simulink model "wlan_phy_rx_pmd"  (Simulink 7.8)
settings: Solver=VariableStepDiscrete  SolverMode=SingleTasking  StartTime=0.0  StopTime=rx_sim.sim_time  RelTol=1e-3
PostLoadFcn:
  | wlan_phy_rx_init
InitFcn:
  | wlan_phy_rx_init
StartFcn:
  | wlan_phy_rx_init
values from neighbouring files (symbols this model uses):
  barker_seq20 = 0.95 * circshift(barker_seq20, [0 4]) ./ max(abs(barker_seq20))    [wlan_phy_rx_init.m]
  bit_scrambler_lfsr_bytes = sum(bit_scrambler_lfsr_bytes .* repmat(2.^[0:7], 127, 1),2)    [wlan_phy_rx_init.m]
  longCorr_coef_i = [3*real(longCorr_coef)]    [wlan_phy_rx_init.m]
  longCorr_coef_q = [3*imag(longCorr_coef)]    [wlan_phy_rx_init.m]
  MAX_CP_LEN = 32    [wlan_phy_rx_init.m]
  MAX_NCBPS = NCBPS_11n_64QAM    (= 312)    [wlan_phy_rx_init.m]
  MAX_NUM_BYTES = 2^16-1    (= 65535)    [wlan_phy_rx_init.m]
  MAX_NUM_SC = 64    [wlan_phy_rx_init.m]
  MAX_NUM_SYMS = 600    [wlan_phy_rx_init.m]
  NDBPS_11a_BPSK12 = 24    [wlan_phy_rx_init.m]
  preFFT_sampBuff_numSamps = 4*MAX_NUM_SC    (= 256)    [wlan_phy_rx_init.m]
  rx_sim = struct()    [wlan_phy_rx_init.m]
  scr_ind_rev = zeros(1,128)    [wlan_phy_rx_init.m]
undefined symbols (not defined in the file or in its folder): gcbh, mcs_rom_11ag, mcs_rom_11n, wlan_rx_deinterleave_rom_gen, xlBlockLoadCallback, xlGetNormalizedPeriod, xlipgetpartsetting
libraries: xbsIndex_r4 x4943, xbsEDKLib_r4 x1
(part 12 of 28)

== system wlan_phy_rx_pmd/FCS & Pkt Buf/Pkt Buf Interface/BRAM IF 64b ==
  note: Word Addr
      | (1K words max)
  "byte " : Inport
  byte_valid : Inport  Port=2
  byte_ind : Inport  Port=3
  Pkt Buf Sel : Inport  Port=4
  9LSB + 3 : xbsIndex_r4/Slice  nbits=9  boolean_output=off  mode=Lower Bit Location + Width  bit1=2  base1=MSB of Input  bit0=3  base0=LSB of Input  dbl_ovrd=off  block_type=slice
      settings: block_version=8.2.02
  AddSub1 : xbsIndex_r4/AddSub  mode=Addition  use_carryin=off  use_carryout=off  en=off  latency=0  precision=Full  arith_type=Unsigned  n_bits=15  bin_pt=0  quantization=Truncate  overflow=Wrap  dbl_ovrd=off  use_behavioral_HDL=off  hw_selection=Fabric  pipelined=off  block_type=addsub
  AddSub2 : xbsIndex_r4/AddSub  mode=Addition  use_carryin=off  use_carryout=off  en=off  latency=0  precision=Full  arith_type=Unsigned  n_bits=15  bin_pt=0  quantization=Truncate  overflow=Wrap  dbl_ovrd=off  use_behavioral_HDL=off  hw_selection=Fabric  pipelined=off  block_type=addsub
  BRAM I/O : Subsystem
  BRAM WEn Decoding : Subsystem
  Concat : xbsIndex_r4/Concat  num_inputs=4  dbl_ovrd=off  block_type=concat
      settings: block_version=8.2.02
  Concat1 : xbsIndex_r4/Concat  num_inputs=4  dbl_ovrd=off  block_type=concat
      settings: block_version=8.2.02
  Concat{2..4,6..7} (5 alike) : xbsIndex_r4/Concat  num_inputs=2  dbl_ovrd=off  block_type=concat
      settings: block_version=8.2.02
  Concat5 : xbsIndex_r4/Concat  num_inputs=4  dbl_ovrd=off  block_type=concat
      settings: block_version=8.2.02
  Constant1 : xbsIndex_r4/Constant  const=0  gui_display_data_type=Fixed-point  arith_type=Unsigned  n_bits=2  bin_pt=0  preci_type=Single  exp_width=8  frac_width=24  explicit_period=off  period=1  equ=P=C  opselect=C  inp2=PCIN>>17  opr=+  inp1=P  carry=CIN  dbl_ovrd=off  block_type=constant
  Constant2 : xbsIndex_r4/Constant  const=0  gui_display_data_type=Fixed-point  arith_type=Unsigned  n_bits=4  bin_pt=0  preci_type=Single  exp_width=8  frac_width=24  explicit_period=off  period=1  equ=P=C  opselect=C  inp2=PCIN>>17  opr=+  inp1=P  carry=CIN  dbl_ovrd=off  block_type=constant
  Constant3 : xbsIndex_r4/Constant  const=0  gui_display_data_type=Fixed-point  arith_type=Unsigned  n_bits=16  bin_pt=0  preci_type=Single  exp_width=8  frac_width=24  explicit_period=on  period=1  equ=P=C  opselect=C  inp2=PCIN>>17  opr=+  inp1=P  carry=CIN  dbl_ovrd=off  block_type=constant
      settings: block_version=8.2.02
  Constant4 : xbsIndex_r4/Constant  const=0  gui_display_data_type=Fixed-point  arith_type=Unsigned  n_bits=3  bin_pt=0  preci_type=Single  exp_width=8  frac_width=24  explicit_period=off  period=1  equ=P=C  opselect=C  inp2=PCIN>>17  opr=+  inp1=P  carry=CIN  dbl_ovrd=off  block_type=constant
  Constant5 : xbsIndex_r4/Constant  const=0  gui_display_data_type=Fixed-point  arith_type=Unsigned  n_bits=3  bin_pt=0  preci_type=Single  exp_width=8  frac_width=24  explicit_period=on  period=1  equ=P=C  opselect=C  inp2=PCIN>>17  opr=+  inp1=P  carry=CIN  dbl_ovrd=off  block_type=constant
      settings: block_version=8.2.02
  Constant7 : xbsIndex_r4/Constant  const=0  gui_display_data_type=Fixed-point  arith_type=Unsigned  n_bits=3  bin_pt=0  preci_type=Single  exp_width=8  frac_width=24  explicit_period=off  period=1  equ=P=C  opselect=C  inp2=PCIN>>17  opr=+  inp1=P  carry=CIN  dbl_ovrd=off  block_type=constant
  Convert : xbsIndex_r4/Convert  gui_display_data_type=Fixed-point  arith_type=Unsigned  n_bits=8  bin_pt=0  float_type=Single  exp_bits=8  fraction_bits=24  quantization=Truncate  overflow=Wrap  en=off  latency=0  dbl_ovrd=off  pipeline=off  block_type=convert
  Display : Display
  Gateway Out{1..12} (12 alike) : xbsIndex_r4/Gateway Out  inherit_from_input=off  hdl_port=off  timing_constraint=None  locs_specified=off  LOCs={}  UseAsDAC=off  DACChannel='1'  block_type=gatewayout
  LSB : xbsIndex_r4/Slice  nbits=1  boolean_output=off  mode=Lower Bit Location + Width  bit1=0  base1=MSB of Input  bit0=0  base0=LSB of Input  dbl_ovrd=off  block_type=slice
      settings: block_version=8.2.02
  Logical : xbsIndex_r4/Logical  logical_function=AND  inputs=2  en=off  latency=0  precision=Full  arith_type=Unsigned  n_bits=16  bin_pt=0  align_bp=on  dbl_ovrd=off  block_type=logical
      settings: block_version=8.2.02
  Logical4 : xbsIndex_r4/Logical  logical_function=XOR  inputs=2  en=off  latency=0  precision=Full  arith_type=Unsigned  n_bits=16  bin_pt=0  align_bp=on  dbl_ovrd=off  block_type=logical
  Mux{,1..3} (4 alike) : xbsIndex_r4/Mux  inputs=2  en=off  latency=0  precision=Full  arith_type=Unsigned  n_bits=16  bin_pt=14  quantization=Truncate  overflow=Wrap  dbl_ovrd=off  block_type=mux
  Pkt Buf Addr : Scope  NumInputPorts=8
      settings: TimeRange=10000  YMin=0~0~0~0~0~-1~-1~-1  YMax=17.5~150~17.5~1~4.5e+009~1~1~1  SaveName=ScopeData27
  To Workspace : ToWorkspace  VariableName=pkt_buf_bram_data  SampleTime=-1  SaveFormat=Array
  To Workspace1 : ToWorkspace  VariableName=pkt_buf_bram_addr  SampleTime=-1  SaveFormat=Array
  To Workspace2 : ToWorkspace  VariableName=pkt_buf_bram_wen  SampleTime=-1  SaveFormat=Array
  wires:
  Gateway Out4/1 -> Pkt Buf Addr/4  "Rx Byte Valid"
  9LSB + 3/1 -> Concat1/3
  byte_valid/1 -> BRAM WEn Decoding/2(byte_valid), Gateway Out4/1
  AddSub2/1 -> Mux1/2, BRAM WEn Decoding/1(byte_addr), Gateway Out2/1
  Pkt Buf Sel/1 -> Concat1/2
  "byte "/1 -> Concat/1, Concat/2, Concat/3, Concat/4
  Gateway Out2/1 -> Pkt Buf Addr/3  "Rx Byte Index"
  Gateway Out1/1 -> Pkt Buf Addr/2  "BRAM WEN"
  Gateway Out10/1 -> Pkt Buf Addr/1  "BRAM Addr"
  Constant3/1 -> Concat1/1
  Constant5/1 -> Concat1/4
  Concat1/1 -> BRAM I/O/2(Addr[31:0]), Gateway Out10/1, Gateway Out11/1
  BRAM WEn Decoding/1(WrEn[7:0]) -> Mux/2
  byte_ind/1 -> AddSub2/1
  Concat2/1 -> AddSub2/2
  [regRx_PktBuf_Rx_Addr_Offset] -> Concat2/1
  Constant7/1 -> Concat2/2
  Gateway Out3/1 -> Pkt Buf Addr/8  "H Est Valid"
  Mux2/1 -> Concat3/1, Concat3/2, Gateway Out7/1, Gateway Out9/1
  Concat/1 -> Mux2/2
  [CHAN_EST_IND] -> Concat4/1, LSB/1, Gateway Out5/1
  Constant1/1 -> Concat4/2
  Mux1/1 -> 9LSB + 3/1
  Logical/1 -> Mux2/1, Mux1/1, Mux/1
  Mux3/1 -> Mux/3, Gateway Out6/1
  [CHAN_EST_VALID] -> Concat5/1, Concat5/2, Concat5/3, Concat5/4, Gateway Out3/1
  Concat5/1 -> Concat6/1, Convert/1
  Constant2/1 -> Concat6/2
  [regRx_Record_Chan_Est_Pkt_Buf] -> Logical/1
  [CHAN_EST_VALID] -> Logical/2
  Concat4/1 -> AddSub1/2
  Concat7/1 -> AddSub1/1
  [regRx_PktBuf_Rx_H_Est_Offset] -> Concat7/1, Gateway Out8/1
  Constant4/1 -> Concat7/2
  AddSub1/1 -> Mux1/3
  Concat3/1 -> BRAM I/O/1(Dout[63:0])
  Mux/1 -> BRAM I/O/3(WEn[7:0]), Gateway Out1/1, Gateway Out12/1
  Concat6/1 -> Mux3/3
  Convert/1 -> Mux3/2
  [regRx_PKT_BUF_H_EST_ORDER_SWAP] -> Logical4/1
  LSB/1 -> Logical4/2
  Logical4/1 -> Mux3/1
  Gateway Out5/1 -> Pkt Buf Addr/7  "H Est Ind"
  Gateway Out6/1 -> Pkt Buf Addr/6  "H Est WrEn"
  [CHAN_EST_IQ_32b] -> Mux2/3
  Gateway Out7/1 -> Pkt Buf Addr/5  "Dout 32"
  Gateway Out8/1 -> Display/1
  Gateway Out11/1 -> To Workspace1/1
  Gateway Out9/1 -> To Workspace/1
  Gateway Out12/1 -> To Workspace2/1

== system wlan_phy_rx_pmd/FCS & Pkt Buf/Pkt Buf Interface/DSSS Addr Calc ==
  note: DSSS Byte Index Rx -> Memory mapping
      |
      | R=Rx byte index
      | M=Memory byte index
      |
      | R  M
      | 0  0   SIGNAL[0]
      | 1  1   SERVICE[0]
      | 2  2   LENGTH[0]
      | 3  3   LENGTH[1]
      | 4  4   CRC16[0]
      | 5  5   CRC16[1]
      |    6   no write
      |   ...
      |    15  no write
      | 6  16  MPDU[0]
      | 7  17  MPDU[1]
      | 8  18  MPDU[2]
      | ...
  R : Inport
  AddSub1 : xbsIndex_r4/AddSub  mode=Addition  use_carryin=off  use_carryout=off  en=off  latency=0  precision=Full  arith_type=Unsigned  n_bits=3  bin_pt=0  quantization=Truncate  overflow=Wrap  dbl_ovrd=off  use_behavioral_HDL=off  hw_selection=Fabric  pipelined=off  block_type=addsub
  Constant1 : xbsIndex_r4/Constant  const=5  gui_display_data_type=Fixed-point  arith_type=Unsigned  n_bits=3  bin_pt=0  preci_type=Single  exp_width=8  frac_width=24  explicit_period=off  period=1  equ=P=C  opselect=C  inp2=PCIN>>17  opr=+  inp1=P  carry=CIN  dbl_ovrd=off  block_type=constant
  Constant3 : xbsIndex_r4/Constant  const=10  gui_display_data_type=Fixed-point  arith_type=Unsigned  n_bits=4  bin_pt=0  preci_type=Single  exp_width=8  frac_width=24  explicit_period=off  period=1  equ=P=C  opselect=C  inp2=PCIN>>17  opr=+  inp1=P  carry=CIN  dbl_ovrd=off  block_type=constant
  Mux5 : xbsIndex_r4/Mux  inputs=2  en=off  latency=0  precision=Full  arith_type=Unsigned  n_bits=16  bin_pt=14  quantization=Truncate  overflow=Wrap  dbl_ovrd=off  block_type=mux
  Relational : xbsIndex_r4/Relational  mode=a<=b  en=off  latency=0  dbl_ovrd=off  block_type=relational
  M : Outport
  wires:
  Mux5/1 -> M/1
  Constant1/1 -> Relational/2
  Relational/1 -> Mux5/1
  R/1 -> Relational/1, AddSub1/1, Mux5/3
  Constant3/1 -> AddSub1/2
  AddSub1/1 -> Mux5/2

== system wlan_phy_rx_pmd/FCS & Pkt Buf/Pkt Buf Interface/OFDM Addr Calc ==
  note: OFDM Byte Index Rx -> Memory mapping
      | 11a/g Rx
      |
      | R=Rx byte index
      | M=Memory byte index
      |
      | R  M
      | 0  0   SIGNAL[0]
      | 1  1   SIGNAL[1]
      | 2  2   SIGNAL[2]
      | 3  3   SERVICE[0]
      | 4  4   SERVICE[1]
      |    5   no write
      |    ...
      |    15   no write
      | 5  16  MPDU[0]
      | 6  17  MPDU[1]
      | ...
  note: OFDM Byte Index Rx -> Memory mapping
      | 11n/ac Rx
      |
      | R=Rx byte index
      | M=Memory byte index
      |
      | R  M
      | 0  0   SIGNAL[0]
      | 1  1   SIGNAL[1]
      | 2  2   SIGNAL[2]
      | 3  3   HT-SIG[0]
      | 4  4   HT-SIG[1]
      | 5  5   HT-SIG[2]
      | 6  6   HT-SIG[3]
      | 7  7   HT-SIG[4]
      | 8  8   HT-SIG[5]
      | 9  9   SERVICE[0]
      | 10 10  SERVICE[1]
      |    11  no write
      | ...
      |    15  no write
      | 11 16  MAC Payload[0]
      | 12 17  MAC Payload[1]
      | ...
  R : Inport
  AddSub1 : xbsIndex_r4/AddSub  mode=Addition  use_carryin=off  use_carryout=off  en=off  latency=0  precision=Full  arith_type=Unsigned  n_bits=3  bin_pt=0  quantization=Truncate  overflow=Wrap  dbl_ovrd=off  use_behavioral_HDL=off  hw_selection=Fabric  pipelined=off  block_type=addsub
  Constant1 : xbsIndex_r4/Constant  const=4  gui_display_data_type=Fixed-point  arith_type=Unsigned  n_bits=3  bin_pt=0  preci_type=Single  exp_width=8  frac_width=24  explicit_period=off  period=1  equ=P=C  opselect=C  inp2=PCIN>>17  opr=+  inp1=P  carry=CIN  dbl_ovrd=off  block_type=constant
  Constant2 : xbsIndex_r4/Constant  const=11  gui_display_data_type=Fixed-point  arith_type=Unsigned  n_bits=4  bin_pt=0  preci_type=Single  exp_width=8  frac_width=24  explicit_period=off  period=1  equ=P=C  opselect=C  inp2=PCIN>>17  opr=+  inp1=P  carry=CIN  dbl_ovrd=off  block_type=constant
  Constant3 : xbsIndex_r4/Constant  const=10  gui_display_data_type=Fixed-point  arith_type=Unsigned  n_bits=4  bin_pt=0  preci_type=Single  exp_width=8  frac_width=24  explicit_period=off  period=1  equ=P=C  opselect=C  inp2=PCIN>>17  opr=+  inp1=P  carry=CIN  dbl_ovrd=off  block_type=constant
  Constant4 : xbsIndex_r4/Constant  const=5  gui_display_data_type=Fixed-point  arith_type=Unsigned  n_bits=3  bin_pt=0  preci_type=Single  exp_width=8  frac_width=24  explicit_period=off  period=1  equ=P=C  opselect=C  inp2=PCIN>>17  opr=+  inp1=P  carry=CIN  dbl_ovrd=off  block_type=constant
  Mux1 : xbsIndex_r4/Mux  inputs=2  en=off  latency=0  precision=Full  arith_type=Unsigned  n_bits=16  bin_pt=14  quantization=Truncate  overflow=Wrap  dbl_ovrd=off  block_type=mux
  Mux2 : xbsIndex_r4/Mux  inputs=2  en=off  latency=0  precision=Full  arith_type=Unsigned  n_bits=16  bin_pt=14  quantization=Truncate  overflow=Wrap  dbl_ovrd=off  block_type=mux
  Mux5 : xbsIndex_r4/Mux  inputs=2  en=off  latency=0  precision=Full  arith_type=Unsigned  n_bits=16  bin_pt=14  quantization=Truncate  overflow=Wrap  dbl_ovrd=off  block_type=mux
  Relational : xbsIndex_r4/Relational  mode=a<=b  en=off  latency=0  dbl_ovrd=off  block_type=relational
  M : Outport
  wires:
  Mux2/1 -> AddSub1/2
  R/1 -> Relational/1, AddSub1/1, Mux5/3
  Relational/1 -> Mux5/1
  Mux5/1 -> M/1
  AddSub1/1 -> Mux5/2
  [RX_PHY_MODE_11N_AC] -> Mux1/1, Mux2/1
  Constant1/1 -> Mux1/2
  Mux1/1 -> Relational/2
  Constant2/1 -> Mux2/2
  Constant3/1 -> Mux1/3
  Constant4/1 -> Mux2/3

== system wlan_phy_rx_pmd/FCS & Pkt Buf/Pkt Buf Interface/Rx Data MAC Outputs ==
  note: Byte index output to MAC is
      | indexed so 0 = MPDU start
      | (0x10 into pkt buffer)
  note: Outputs to MAC hw
  "Byte " : Inport
  Byte Valid : Inport  Port=2
  Byte Ind : Inport  Port=3
  AddSub1 : xbsIndex_r4/AddSub  mode=Subtraction  use_carryin=off  use_carryout=off  en=off  latency=0  precision=User Defined  arith_type=Unsigned  n_bits=ceil(log2(MAX_NUM_BYTES))  bin_pt=0  quantization=Truncate  overflow=Saturate  dbl_ovrd=off  use_behavioral_HDL=off  hw_selection=Fabric  pipelined=off  block_type=addsub
  Constant4 : xbsIndex_r4/Constant  const=16  gui_display_data_type=Fixed-point  arith_type=Unsigned  n_bits=5  bin_pt=0  preci_type=Single  exp_width=8  frac_width=24  explicit_period=off  period=1  equ=P=C  opselect=C  inp2=PCIN>>17  opr=+  inp1=P  carry=CIN  dbl_ovrd=off  block_type=constant
  Logical4 : xbsIndex_r4/Logical  logical_function=AND  inputs=2  en=off  latency=0  precision=Full  arith_type=Unsigned  n_bits=16  bin_pt=0  align_bp=on  dbl_ovrd=off  block_type=logical
  Relational2 : xbsIndex_r4/Relational  mode=a>=b  en=off  latency=0  dbl_ovrd=off  block_type=relational
  tag scope: CUR_MPDU_BYTE=global, CUR_MPDU_BYTE_INDEX=global, CUR_MPDU_BYTE_VALID=global
  wires:
  Logical4/1 -> [CUR_MPDU_BYTE_VALID]
  Relational2/1 -> Logical4/1
  AddSub1/1 -> [CUR_MPDU_BYTE_INDEX]
  Constant4/1 -> AddSub1/2, Relational2/2
  Byte Ind/1 -> AddSub1/1, Relational2/1
  Byte Valid/1 -> Logical4/2
  "Byte "/1 -> [CUR_MPDU_BYTE]

== system wlan_phy_rx_pmd/FFT/Reset Gen/S-R Latch1 ==
  S : Inport
  R : Inport  Port=2
  Constant1 : xbsIndex_r4/Constant  const=1  gui_display_data_type=Fixed-point  arith_type=Boolean  n_bits=1  bin_pt=0  preci_type=Single  exp_width=8  frac_width=24  explicit_period=off  period=1  equ=P=C  opselect=C  inp2=PCIN>>17  opr=+  inp1=P  carry=CIN  dbl_ovrd=off  block_type=constant
      settings: block_version=VER_STRING_GOES_HERE
  Convert1 : xbsIndex_r4/Convert  gui_display_data_type=Boolean  arith_type=Boolean  n_bits=1  bin_pt=0  float_type=Single  exp_bits=8  fraction_bits=24  quantization=Truncate  overflow=Wrap  en=off  latency=0  dbl_ovrd=off  pipeline=off  block_type=convert
  Convert2 : xbsIndex_r4/Convert  gui_display_data_type=Boolean  arith_type=Boolean  n_bits=1  bin_pt=0  float_type=Single  exp_bits=8  fraction_bits=24  quantization=Truncate  overflow=Wrap  en=off  latency=0  dbl_ovrd=off  pipeline=off  block_type=convert
  Register2 : xbsIndex_r4/Register  init=0  rst=on  en=on  dbl_ovrd=off  block_type=register
      settings: xl_area=[1 1 0 0 0 0 0]  block_version=VER_STRING_GOES_HERE
  Q : Outport
  wires:
  S/1 -> Convert2/1
  Register2/1 -> Q/1
  R/1 -> Convert1/1
  Constant1/1 -> Register2/1
  Convert1/1 -> Register2/2
  Convert2/1 -> Register2/3

== system wlan_phy_rx_pmd/FFT/Sym Count/S-R Latch1 ==
  S : Inport
  R : Inport  Port=2
  Constant1 : xbsIndex_r4/Constant  const=1  gui_display_data_type=Fixed-point  arith_type=Boolean  n_bits=1  bin_pt=0  preci_type=Single  exp_width=8  frac_width=24  explicit_period=off  period=1  equ=P=C  opselect=C  inp2=PCIN>>17  opr=+  inp1=P  carry=CIN  dbl_ovrd=off  block_type=constant
      settings: block_version=VER_STRING_GOES_HERE
  Convert1 : xbsIndex_r4/Convert  gui_display_data_type=Boolean  arith_type=Boolean  n_bits=1  bin_pt=0  float_type=Single  exp_bits=8  fraction_bits=24  quantization=Truncate  overflow=Wrap  en=off  latency=0  dbl_ovrd=off  pipeline=off  block_type=convert
  Convert2 : xbsIndex_r4/Convert  gui_display_data_type=Boolean  arith_type=Boolean  n_bits=1  bin_pt=0  float_type=Single  exp_bits=8  fraction_bits=24  quantization=Truncate  overflow=Wrap  en=off  latency=0  dbl_ovrd=off  pipeline=off  block_type=convert
  Register2 : xbsIndex_r4/Register  init=0  rst=on  en=on  dbl_ovrd=off  block_type=register
      settings: xl_area=[1 1 0 0 0 0 0]  block_version=VER_STRING_GOES_HERE
  Q : Outport
  wires:
  S/1 -> Convert2/1
  Register2/1 -> Q/1
  R/1 -> Convert1/1
  Constant1/1 -> Register2/1
  Convert1/1 -> Register2/2
  Convert2/1 -> Register2/3

== system wlan_phy_rx_pmd/Inputs/RF A Inputs/RF A RSSI Sum ==
  RSSI : Inport
  Down Sample6 : xbsIndex_r4/Down Sample  sample_ratio=16  sample_phase="Last Value of Frame  (most efficient)"  en=off  latency=1  dbl_ovrd=off  block_type=dsamp
      settings: block_version=VER_STRING_GOES_HERE
  RSSI DET Debug Out : Subsystem
  Running Sum : Subsystem
  Sum : Outport
  wires:
  [RX_GLOBAL_RESET] -> Down Sample6/1
  RSSI/1 -> Running Sum/1(RSSI), RSSI DET Debug Out/2(Raw RSSI)
  Down Sample6/1 -> Running Sum/2(reset)
  Running Sum/1(avg) -> Sum/1, RSSI DET Debug Out/1(Avg RSSI)

== system wlan_phy_rx_pmd/Inputs/RF B Inputs/RF B RSSI Sum ==
  RSSI : Inport
  Down Sample6 : xbsIndex_r4/Down Sample  sample_ratio=16  sample_phase="Last Value of Frame  (most efficient)"  en=off  latency=1  dbl_ovrd=off  block_type=dsamp
      settings: block_version=VER_STRING_GOES_HERE
  Running Sum : Subsystem
  Sum : Outport
  wires:
  Running Sum/1(avg) -> Sum/1
  Down Sample6/1 -> Running Sum/2(reset)
  RSSI/1 -> Running Sum/1(RSSI)
  [RX_GLOBAL_RESET] -> Down Sample6/1

== system wlan_phy_rx_pmd/Inputs/RF C Inputs/RF C RSSI Sum ==
  RSSI : Inport
  Down Sample6 : xbsIndex_r4/Down Sample  sample_ratio=16  sample_phase="Last Value of Frame  (most efficient)"  en=off  latency=1  dbl_ovrd=off  block_type=dsamp
      settings: block_version=VER_STRING_GOES_HERE
  Running Sum : Subsystem
  Sum : Outport
  wires:
  Running Sum/1(avg) -> Sum/1
  Down Sample6/1 -> Running Sum/2(reset)
  RSSI/1 -> Running Sum/1(RSSI)
  [RX_GLOBAL_RESET] -> Down Sample6/1

== system wlan_phy_rx_pmd/Inputs/RF D Inputs/RF D RSSI Sum ==
  RSSI : Inport
  Down Sample6 : xbsIndex_r4/Down Sample  sample_ratio=16  sample_phase="Last Value of Frame  (most efficient)"  en=off  latency=1  dbl_ovrd=off  block_type=dsamp
      settings: block_version=VER_STRING_GOES_HERE
  Running Sum : Subsystem
  Sum : Outport
  wires:
  [RX_GLOBAL_RESET] -> Down Sample6/1
  RSSI/1 -> Running Sum/1(RSSI)
  Down Sample6/1 -> Running Sum/2(reset)
  Running Sum/1(avg) -> Sum/1

== system wlan_phy_rx_pmd/MAC I/O/Pulse Stretch1/S-R Latch ==
  S : Inport
  R : Inport  Port=2
  Constant1 : xbsIndex_r4/Constant  const=1  gui_display_data_type=Boolean  arith_type=Boolean  n_bits=1  bin_pt=0  preci_type=Single  exp_width=8  frac_width=24  explicit_period=off  period=1  equ=P=C  opselect=C  inp2=PCIN>>17  opr=+  inp1=P  carry=CIN  dbl_ovrd=off  block_type=constant
      settings: block_version=VER_STRING_GOES_HERE
  Convert1 : xbsIndex_r4/Convert  gui_display_data_type=Boolean  arith_type=Boolean  n_bits=1  bin_pt=0  float_type=Single  exp_bits=8  fraction_bits=24  quantization=Truncate  overflow=Wrap  en=off  latency=0  dbl_ovrd=off  pipeline=off  block_type=convert
  Convert2 : xbsIndex_r4/Convert  gui_display_data_type=Boolean  arith_type=Boolean  n_bits=1  bin_pt=0  float_type=Single  exp_bits=8  fraction_bits=24  quantization=Truncate  overflow=Wrap  en=off  latency=0  dbl_ovrd=off  pipeline=off  block_type=convert
  Register2 : xbsIndex_r4/Register  init=0  rst=on  en=on  dbl_ovrd=off  block_type=register
      settings: xl_area=[1 1 0 0 0 0 0]  block_version=VER_STRING_GOES_HERE
  Q : Outport
  wires:
  Convert2/1 -> Register2/3
  Convert1/1 -> Register2/2
  Constant1/1 -> Register2/1
  R/1 -> Convert1/1
  Register2/1 -> Q/1
  S/1 -> Convert2/1

== system wlan_phy_rx_pmd/Sync & Antenna Sel/Antenna Selection/Ant Sel Logic ==
  note: [0 0] = RF A
      | [0 1] = RF B
      | [1 0] = RF C
      | [1 1] = RF D
  note: [1 1] = RF A
      | [1 0] = RF B
      | [0 1] = RF C
      | [0 0] = RF D
  G A : Inport
  G B : Inport  Port=2
  G C : Inport  Port=3
  G D : Inport  Port=4
  Concat : xbsIndex_r4/Concat  num_inputs=2  dbl_ovrd=off  block_type=concat
  Inverter2 : xbsIndex_r4/Inverter  en=off  latency=0  dbl_ovrd=off  block_type=inv
  Mux{,1..3} (4 alike) : xbsIndex_r4/Mux  inputs=2  en=off  latency=0  precision=Full  arith_type=Unsigned  n_bits=16  bin_pt=14  quantization=Truncate  overflow=Wrap  dbl_ovrd=off  block_type=mux
  RF A En Mask : Subsystem
  RF B En Mask : Subsystem
  RF C En Mask : Subsystem
  RF D En Mask : Subsystem
  Relational : xbsIndex_r4/Relational  mode=a<=b  en=off  latency=0  dbl_ovrd=off  block_type=relational
  Relational1 : xbsIndex_r4/Relational  mode=a<=b  en=off  latency=0  dbl_ovrd=off  block_type=relational
  Relational2 : xbsIndex_r4/Relational  mode=a<=b  en=off  latency=0  dbl_ovrd=off  block_type=relational
  Ant Sel : Outport
  wires:
  RF D En Mask/1(" GD") -> Relational1/2, Mux1/2
  RF C En Mask/1(" GC") -> Relational1/1, Mux1/3
  RF B En Mask/1(" GB") -> Relational/2, Mux/2
  RF A En Mask/1(" GA") -> Relational/1, Mux/3
  Relational/1 -> Mux/1, Mux2/3
  Mux/1 -> Relational2/1
  Relational1/1 -> Mux1/1, Mux2/2
  Mux1/1 -> Relational2/2
  Relational2/1 -> Concat/1, Mux2/1
  Mux2/1 -> Concat/2
  Concat/1 -> Inverter2/1
  Inverter2/1 -> Mux3/3
  [regRx_switchingDiv_En] -> Mux3/1
  G A/1 -> RF A En Mask/1(GA)
  G B/1 -> RF B En Mask/1(GB)
  G C/1 -> RF C En Mask/1(GC)
  G D/1 -> RF D En Mask/1(GD)
  [regRx_noSwitching_AntSel] -> Mux3/2
  Mux3/1 -> Ant Sel/1

== system wlan_phy_rx_pmd/Sync & Antenna Sel/Antenna Selection/Posedge ==
  note: Rising Edge Detection
  D : Inport
  Delay : xbsIndex_r4/Delay  rst=off  infoeditControl=Selection of Reset will increase slice count due to use of real FFs and instead of SRLs  en=off  latency=1  dbl_ovrd=off  reg_retiming=off  block_type=delay
      settings: infoedit=Hardware notes: A delay line is a chain, each link of which is an SRL16 followed by a flip-flop. If register retiming is enabled, the delay line is a chain of flip-flops.  xl_area=[1 1 0 0 0 0 0]  block_version=VER_STRING_GOES_HERE
  Inverter : xbsIndex_r4/Inverter  en=off  latency=0  dbl_ovrd=off  block_type=inv
      settings: xl_area=[0 0 0 0 0 0 0]  block_version=VER_STRING_GOES_HERE
  Logical1 : xbsIndex_r4/Logical  logical_function=AND  inputs=2  en=off  latency=0  precision=Full  arith_type=Unsigned  n_bits=8  bin_pt=2  align_bp=on  dbl_ovrd=off  block_type=logical
      settings: xl_area=[1 0 0 1 0 0 0]  block_version=VER_STRING_GOES_HERE
  Q : Outport
  wires:
  D/1 -> Logical1/2, Delay/1
  Logical1/1 -> Q/1
  Inverter/1 -> Logical1/1
  Delay/1 -> Inverter/1

== system wlan_phy_rx_pmd/Sync & Antenna Sel/Antenna Selection/RF A Gains ==
  Capt : Inport
  Rst : Inport  Port=2
  Concat : xbsIndex_r4/Concat  num_inputs=2  dbl_ovrd=off  block_type=concat
  Register2 : xbsIndex_r4/Register  init=0  rst=on  en=on  dbl_ovrd=off  block_type=register
      settings: xl_area=[1 1 0 0 0 0 0]  block_version=VER_STRING_GOES_HERE
  G_vec : Outport
  wires:
  [RFA_AGC_G_RF] -> Concat/1
  [RFA_AGC_G_BB] -> Concat/2
  Concat/1 -> Register2/1
  Rst/1 -> Register2/2
  Capt/1 -> Register2/3
  Register2/1 -> G_vec/1

== system wlan_phy_rx_pmd/Sync & Antenna Sel/Antenna Selection/RF B Gains ==
  Capt : Inport
  Rst : Inport  Port=2
  Concat : xbsIndex_r4/Concat  num_inputs=2  dbl_ovrd=off  block_type=concat
  Register2 : xbsIndex_r4/Register  init=0  rst=on  en=on  dbl_ovrd=off  block_type=register
      settings: xl_area=[1 1 0 0 0 0 0]  block_version=VER_STRING_GOES_HERE
  G_vec : Outport
  wires:
  Register2/1 -> G_vec/1
  Capt/1 -> Register2/3
  Rst/1 -> Register2/2
  Concat/1 -> Register2/1
  [RFB_AGC_G_BB] -> Concat/2
  [RFB_AGC_G_RF] -> Concat/1

== system wlan_phy_rx_pmd/Sync & Antenna Sel/Antenna Selection/RF C Gains ==
  Capt : Inport
  Rst : Inport  Port=2
  Concat : xbsIndex_r4/Concat  num_inputs=2  dbl_ovrd=off  block_type=concat
  Register2 : xbsIndex_r4/Register  init=0  rst=on  en=on  dbl_ovrd=off  block_type=register
      settings: xl_area=[1 1 0 0 0 0 0]  block_version=VER_STRING_GOES_HERE
  G_vec : Outport
  wires:
  Register2/1 -> G_vec/1
  Capt/1 -> Register2/3
  Rst/1 -> Register2/2
  Concat/1 -> Register2/1
  [RFC_AGC_G_BB] -> Concat/2
  [RFC_AGC_G_RF] -> Concat/1

== system wlan_phy_rx_pmd/Sync & Antenna Sel/Antenna Selection/RF D Gains ==
  Capt : Inport
  Rst : Inport  Port=2
  Concat : xbsIndex_r4/Concat  num_inputs=2  dbl_ovrd=off  block_type=concat
  Register2 : xbsIndex_r4/Register  init=0  rst=on  en=on  dbl_ovrd=off  block_type=register
      settings: xl_area=[1 1 0 0 0 0 0]  block_version=VER_STRING_GOES_HERE
  G_vec : Outport
  wires:
  [RFD_AGC_G_RF] -> Concat/1
  [RFD_AGC_G_BB] -> Concat/2
  Concat/1 -> Register2/1
  Rst/1 -> Register2/2
  Capt/1 -> Register2/3
  Register2/1 -> G_vec/1

== system wlan_phy_rx_pmd/Sync & Antenna Sel/Antenna Selection/RSSI & Gain Capture ==
  note: Capture RSSI after AGC has settled
      | (avoids saturating RSSI when G_RF=3 and Rx power is high)
      | Software must compensate for RF gain selection when
      | interpretting thse RSSI values.
  note: Capture gains for all antennas
      | after AGC has settled.
  RSSI Vec : Inport
  16MSB{,1..3} (4 alike) : xbsIndex_r4/Slice  nbits=16  boolean_output=off  mode=Upper Bit Location + Width  bit1=0  base1=MSB of Input  bit0=0  base0=LSB of Input  dbl_ovrd=off  block_type=slice
  Bus Selector3 : BusSelector  OutputSignals=RFB_RSSI,RFA_RSSI,RFD_RSSI,RFC_RSSI
  Concat{,2..4,6..7} (6 alike) : xbsIndex_r4/Concat  num_inputs=2  dbl_ovrd=off  block_type=concat
  Concat1 : xbsIndex_r4/Concat  num_inputs=7  dbl_ovrd=off  block_type=concat
  Constant1 : xbsIndex_r4/Constant  const=0  gui_display_data_type=Fixed-point  arith_type=Unsigned  n_bits=1  bin_pt=0  preci_type=Single  exp_width=8  frac_width=24  explicit_period=off  period=1  equ=P=C  opselect=C  inp2=PCIN>>17  opr=+  inp1=P  carry=CIN  dbl_ovrd=off  block_type=constant
      settings: block_version=VER_STRING_GOES_HERE
  Delay1 : xbsIndex_r4/Delay  rst=off  infoeditControl=Selection of Reset will increase slice count due to use of real FFs and instead of SRLs  en=off  latency=4  dbl_ovrd=off  reg_retiming=off  block_type=delay
  Posedge1 : Subsystem
  Register1 : xbsIndex_r4/Register  init=0  rst=off  en=on  dbl_ovrd=off  block_type=register
  Register2 : xbsIndex_r4/Register  init=0  rst=off  en=on  dbl_ovrd=off  block_type=register
  Register{3,5..9} (6 alike) : xbsIndex_r4/Register  init=0  rst=off  en=off  dbl_ovrd=off  block_type=register
  Register4 : xbsIndex_r4/Register  init=0  rst=off  en=on  dbl_ovrd=off  block_type=register
  Up Sample : xbsIndex_r4/Up Sample  sample_ratio=16  copy_samples=on  en=off  latency=0  dbl_ovrd=off  block_type=usamp
  Up Sample1 : xbsIndex_r4/Up Sample  sample_ratio=16  copy_samples=on  en=off  latency=0  dbl_ovrd=off  block_type=usamp
  tag scope: GAINS_POST_AGC=global, RSSI_POST_AGC_AB=global, RSSI_POST_AGC_CD=global
  wires:
  [RFA_AGC_G_BB] -> Concat/2
  Register4/1 -> Register5/1
  Up Sample1/1 -> Register4/1
  Concat4/1 -> Up Sample1/1
  Concat6/1 -> Concat1/3
  Concat7/1 -> Concat1/1
  [RFD_AGC_G_RF] -> Concat7/1
  [RFD_AGC_G_BB] -> Concat7/2
  [RFC_AGC_G_BB] -> Concat6/2
  [RFC_AGC_G_RF] -> Concat6/1
  Concat3/1 -> Concat1/5
  Concat/1 -> Concat1/7
  Constant1/1 -> Concat1/6, Concat1/4, Concat1/2
  [RFB_AGC_G_RF] -> Concat3/1
  [RFB_AGC_G_BB] -> Concat3/2
  [RFA_AGC_G_RF] -> Concat/1
  Posedge1/1(Q) -> Delay1/1
  [AGC_DONE] -> Posedge1/1(D)
  16MSB1/1 -> Concat2/2
  16MSB/1 -> Concat2/1
  Up Sample/1 -> Register1/1
  Concat2/1 -> Up Sample/1
  Register1/1 -> Register3/1
  Register2/1 -> Register6/1
  Concat1/1 -> Register2/1
  RSSI Vec/1 -> Bus Selector3/1
  16MSB2/1 -> Concat4/1
  16MSB3/1 -> Concat4/2
  Delay1/1 -> Register1/2, Register4/2, Register2/2
  Bus Selector3/1 -> 16MSB/1  "<RFB_RSSI>"
  Bus Selector3/2 -> 16MSB1/1  "<RFA_RSSI>"
  Bus Selector3/3 -> 16MSB2/1  "<RFD_RSSI>"
  Bus Selector3/4 -> 16MSB3/1  "<RFC_RSSI>"
  Register3/1 -> Register9/1
  Register5/1 -> Register8/1
  Register6/1 -> Register7/1
  Register7/1 -> [GAINS_POST_AGC]
  Register8/1 -> [RSSI_POST_AGC_CD]
  Register9/1 -> [RSSI_POST_AGC_AB]

== system wlan_phy_rx_pmd/Sync & Antenna Sel/Debug  Outputs/Pulse Stretch ==
  P : Inport
  Delay : xbsIndex_r4/Delay  rst=off  infoeditControl=Selection of Reset will increase slice count due to use of real FFs and instead of SRLs  en=off  latency=16  dbl_ovrd=off  reg_retiming=off  block_type=delay
  S-R Latch : Subsystem
  P16 : Outport
  wires:
  S-R Latch/1(Q) -> Delay/1, P16/1
  Delay/1 -> S-R Latch/2(R)
  P/1 -> S-R Latch/1(S)

== system wlan_phy_rx_pmd/Sync & Antenna Sel/Debug  Outputs/Pulse Stretch1 ==
  P : Inport
  Delay : xbsIndex_r4/Delay  rst=off  infoeditControl=Selection of Reset will increase slice count due to use of real FFs and instead of SRLs  en=off  latency=16  dbl_ovrd=off  reg_retiming=off  block_type=delay
  S-R Latch : Subsystem
  P16 : Outport
  wires:
  S-R Latch/1(Q) -> Delay/1, P16/1
  Delay/1 -> S-R Latch/2(R)
  P/1 -> S-R Latch/1(S)

== system wlan_phy_rx_pmd/Sync & Antenna Sel/LTS Correlation/Correlator ==
  note: The architecture for this correlator is based on one developed by Dr. Chris Dick (Cheif DSP Scientist at Xilinx).
      | The version here has been modified in a few ways:
      | -The inner correlators run at 8x the input sample rate (vs 5 in the original)
      | -The "multiplications" are 3-bit by 1-bit (vs 1x1 in the original), which improves
      | correlation performance with interference or overlapping preambles
  note: i
      | q
      | q
      | i
  Rx I : Inport
  Rx Q : Inport  Port=2
  rx_IQ_valid : Inport  Port=3
  AddSub{1..4} (4 alike) : xbsIndex_r4/AddSub  mode=Addition  use_carryin=off  use_carryout=off  en=off  latency=1  precision=Full  arith_type=Unsigned  n_bits=8  bin_pt=2  quantization=Truncate  overflow=Wrap  dbl_ovrd=off  use_behavioral_HDL=on  hw_selection=Fabric  pipelined=off  use_rpm=off  block_type=addsub
      settings: xl_area=[4 7 0 6 0 0 0]  block_version=VER_STRING_GOES_HERE
  C1 : Subsystem  mask{N=4, h=longCorr_coef_i(1:32), Ts_In=4}
      mask prompts: N "Coefficients/correlator"; h "Coefficients"; Ts_In "Input sample period"
  C2 : Subsystem  mask{N=4, h=longCorr_coef_i(1:32), Ts_In=4}
      mask prompts: N "Coefficients/correlator"; h "Coefficients"; Ts_In "Input sample period"
  C3 : Subsystem  mask{N=4, h=longCorr_coef_i(33:64), Ts_In=4}
      mask prompts: N "Coefficients/correlator"; h "Coefficients"; Ts_In "Input sample period"
  C4 : Subsystem  mask{N=4, h=longCorr_coef_i(33:64), Ts_In=4}
      mask prompts: N "Coefficients/correlator"; h "Coefficients"; Ts_In "Input sample period"
  C5 : Subsystem  mask{N=4, h=longCorr_coef_q(1:32), Ts_In=4}
      mask prompts: N "Coefficients/correlator"; h "Coefficients"; Ts_In "Input sample period"
  C6 : Subsystem  mask{N=4, h=longCorr_coef_q(33:64), Ts_In=4}
      mask prompts: N "Coefficients/correlator"; h "Coefficients"; Ts_In "Input sample period"
  C7 : Subsystem  mask{N=4, h=longCorr_coef_q(1:32), Ts_In=4}
      mask prompts: N "Coefficients/correlator"; h "Coefficients"; Ts_In "Input sample period"
  C8 : Subsystem  mask{N=4, h=longCorr_coef_q(33:64), Ts_In=4}
      mask prompts: N "Coefficients/correlator"; h "Coefficients"; Ts_In "Input sample period"
  Combine : Subsystem
  Delay1 : xbsIndex_r4/Delay  rst=off  infoeditControl=Selection of Reset will increase slice count due to use of real FFs and instead of SRLs  en=on  latency=1  dbl_ovrd=off  reg_retiming=off  block_type=delay
  Delay2 : xbsIndex_r4/Delay  rst=off  infoeditControl=Selection of Reset will increase slice count due to use of real FFs and instead of SRLs  en=on  latency=1  dbl_ovrd=off  reg_retiming=off  block_type=delay
  Delay3 : xbsIndex_r4/Delay  rst=off  infoeditControl=Selection of Reset will increase slice count due to use of real FFs and instead of SRLs  en=off  latency=1  dbl_ovrd=off  reg_retiming=off  block_type=delay
  Delay4 : xbsIndex_r4/Delay  rst=off  infoeditControl=Selection of Reset will increase slice count due to use of real FFs and instead of SRLs  en=off  latency=1  dbl_ovrd=off  reg_retiming=off  block_type=delay
  FSM : Subsystem  mask{N=4}
      mask prompts: N "Coefficients/correlator"
  Goto Tag Visibility : GotoTagVisibility  GotoTag=ltsCorr_dAddr
  Goto Tag Visibility1 : GotoTagVisibility  GotoTag=ltsCorr_cAddr
  Goto Tag Visibility2 : GotoTagVisibility  GotoTag=ltsCorr_ld
  R : Outport
  tag scope: CS_LTS_Corr=global, ltsCorr_cAddr=scoped, ltsCorr_dAddr=scoped, ltsCorr_ld=scoped
  wires:
  rx_IQ_valid/1 -> Delay1/2, Delay2/2, Delay4/1, Delay3/1
  FSM/3(load) -> [ltsCorr_ld]
  FSM/2(Coef Addr) -> [ltsCorr_cAddr]
  FSM/1(Data Addr) -> [ltsCorr_dAddr]
  Delay2/1 -> C2/1(x), C5/1(x)
  Delay1/1 -> C1/1(x), C7/1(x)
  Combine/1(R) -> R/1, [CS_LTS_Corr]
  Rx I/1 -> Delay1/1
  Rx Q/1 -> Delay2/1
  Delay3/1 -> FSM/1(IQ_Valid)
  Delay4/1 -> C1/2(x_valid), C5/2(x_valid), C2/2(x_valid), C7/2(x_valid)
  C3/1(y) -> AddSub4/2
  C1/1(y) -> AddSub4/1
  C1/3(x_dly) -> C3/1(x)
  C1/4(x_dly_vout) -> C3/2(x_valid)
  C5/1(y) -> AddSub1/1
  C6/1(y) -> AddSub1/2
  C5/3(x_dly) -> C6/1(x)
  C5/4(x_dly_vout) -> C6/2(x_valid)
  C7/1(y) -> AddSub2/1
  C8/1(y) -> AddSub2/2
  C7/3(x_dly) -> C8/1(x)
  C7/4(x_dly_vout) -> C8/2(x_valid)
  C2/1(y) -> AddSub3/1
  C4/1(y) -> AddSub3/2
  C2/3(x_dly) -> C4/1(x)
  C2/4(x_dly_vout) -> C4/2(x_valid)
  AddSub4/1 -> Combine/1(Re*Re)
  AddSub1/1 -> Combine/2(Im*Im)
  AddSub2/1 -> Combine/3(Re*Im)
  AddSub3/1 -> Combine/4(Im*Re)

== system wlan_phy_rx_pmd/Sync & Antenna Sel/LTS Correlation/LTS Corr Timeout ==
  note: LTS corr asserts when timeout counter is around 290, for
      | a perfect Rx waveform with no AGC and auto-corr pkt det.
  Pkt Det : Inport
  IQ Valid : Inport  Port=2
  LTS 1 : Inport  Port=3
  LTS 2 : Inport  Port=4
  Counter : xbsIndex_r4/Counter  cnt_type=Free Running  cnt_to=Inf  operation=Up  start_count=0  cnt_by_val=1  arith_type=Unsigned  n_bits=9  bin_pt=0  load_pin=off  rst=on  en=on  explicit_period=off  period=1  dbl_ovrd=off  use_behavioral_HDL=off  implementation=Fabric  block_type=counter
  Display : Display
  Gateway Out{1..9} (9 alike) : xbsIndex_r4/Gateway Out  inherit_from_input=off  hdl_port=off  timing_constraint=None  locs_specified=off  LOCs={}  UseAsDAC=off  DACChannel='1'  block_type=gatewayout
  Inverter : xbsIndex_r4/Inverter  en=off  latency=0  dbl_ovrd=off  block_type=inv
  LTS Timeout : Scope  NumInputPorts=10
      settings: TimeRange=18000  YMin=0~0~0~0~0~0~0~0~-1~-1  YMax=1~1~1~1~1~600~1~1~1~1  SaveName=ScopeData17
  Logical : xbsIndex_r4/Logical  logical_function=AND  inputs=2  en=off  latency=0  precision=Full  arith_type=Unsigned  n_bits=16  bin_pt=0  align_bp=on  dbl_ovrd=off  block_type=logical
  Logical1 : xbsIndex_r4/Logical  logical_function=NAND  inputs=2  en=off  latency=0  precision=Full  arith_type=Unsigned  n_bits=16  bin_pt=0  align_bp=on  dbl_ovrd=off  block_type=logical
  Logical2 : xbsIndex_r4/Logical  logical_function=AND  inputs=2  en=off  latency=0  precision=Full  arith_type=Unsigned  n_bits=16  bin_pt=0  align_bp=on  dbl_ovrd=off  block_type=logical
  Relational : xbsIndex_r4/Relational  mode=a>b  en=off  latency=0  dbl_ovrd=off  block_type=relational
  S-R Latch : Subsystem
  S-R Latch1 : Subsystem
  done14 : xbsIndex_r4/Gateway Out  inherit_from_input=off  hdl_port=off  timing_constraint=None  locs_specified=off  LOCs={}  UseAsDAC=off  DACChannel='1'  block_type=gatewayout
      settings: infoedit="Gateway out block.  Converts Xilinx fixed point inputs into ouputs of type Simulink integer, double, or fixed point.<P><P>Hardware notes:  In hardware these blocks become top level output ports or are discarded, depending on how they are configured."  xl_area=[0 0 0 0 1 0 0]  block_version=VER_STRING_GOES_HERE
  Timeout : Outport
  wires:
  Logical/1 -> Counter/2
  Pkt Det/1 -> Logical/1, Inverter/1, Gateway Out5/1
  IQ Valid/1 -> Logical/2
  Inverter/1 -> Counter/1, S-R Latch/2(R), S-R Latch1/2(R)
  LTS 1/1 -> S-R Latch/1(S), Gateway Out4/1
  LTS 2/1 -> S-R Latch1/1(S), Gateway Out1/1
  S-R Latch/1(Q) -> Logical1/1, Gateway Out2/1
  S-R Latch1/1(Q) -> Logical1/2, Gateway Out3/1
  Logical1/1 -> Logical2/1, Gateway Out8/1
  Relational/1 -> Logical2/2, Gateway Out9/1
  Logical2/1 -> Timeout/1, Gateway Out7/1
  Counter/1 -> Relational/1, Gateway Out6/1
  [regRx_PktDet_Timout] -> Relational/2, done14/1
  done14/1 -> Display/1
  Gateway Out4/1 -> LTS Timeout/1  "Corr 1"
  Gateway Out2/1 -> LTS Timeout/2  "Corr 1 Latch"
  Gateway Out1/1 -> LTS Timeout/3  "Corr 2"
  Gateway Out3/1 -> LTS Timeout/4  "Corr 2 Latch"
  Gateway Out5/1 -> LTS Timeout/5  "Pkt Det"
  Gateway Out6/1 -> LTS Timeout/6  "Timeout counter"
  Gateway Out7/1 -> LTS Timeout/10  "Timeout"
  Gateway Out8/1 -> LTS Timeout/7  "No Det"
  Gateway Out9/1 -> LTS Timeout/8  "Counter at limit"

== system wlan_phy_rx_pmd/Sync & Antenna Sel/LTS Correlation/Posedge3 ==
  note: Rising Edge Detection
  D : Inport
  Delay : xbsIndex_r4/Delay  rst=off  infoeditControl=Selection of Reset will increase slice count due to use of real FFs and instead of SRLs  en=off  latency=1  dbl_ovrd=off  reg_retiming=off  block_type=delay
      settings: infoedit=Hardware notes: A delay line is a chain, each link of which is an SRL16 followed by a flip-flop. If register retiming is enabled, the delay line is a chain of flip-flops.  xl_area=[1 1 0 0 0 0 0]  block_version=VER_STRING_GOES_HERE
  Inverter : xbsIndex_r4/Inverter  en=off  latency=0  dbl_ovrd=off  block_type=inv
      settings: xl_area=[0 0 0 0 0 0 0]  block_version=VER_STRING_GOES_HERE
  Logical1 : xbsIndex_r4/Logical  logical_function=AND  inputs=2  en=off  latency=0  precision=Full  arith_type=Unsigned  n_bits=8  bin_pt=2  align_bp=on  dbl_ovrd=off  block_type=logical
      settings: xl_area=[1 0 0 1 0 0 0]  block_version=VER_STRING_GOES_HERE
  Q : Outport
  wires:
  D/1 -> Logical1/2, Delay/1
  Logical1/1 -> Q/1
  Inverter/1 -> Logical1/1
  Delay/1 -> Inverter/1

== system wlan_phy_rx_pmd/Sync & Antenna Sel/LTS Correlation/S-R Latch ==
  S : Inport
  R : Inport  Port=2
  Constant1 : xbsIndex_r4/Constant  const=1  gui_display_data_type=Fixed-point  arith_type=Boolean  n_bits=1  bin_pt=0  preci_type=Single  exp_width=8  frac_width=24  explicit_period=off  period=1  equ=P=C  opselect=C  inp2=PCIN>>17  opr=+  inp1=P  carry=CIN  dbl_ovrd=off  block_type=constant
      settings: block_version=VER_STRING_GOES_HERE
  Convert1 : xbsIndex_r4/Convert  gui_display_data_type=Boolean  arith_type=Boolean  n_bits=1  bin_pt=0  float_type=Single  exp_bits=8  fraction_bits=24  quantization=Truncate  overflow=Wrap  en=off  latency=0  dbl_ovrd=off  pipeline=off  block_type=convert
  Convert2 : xbsIndex_r4/Convert  gui_display_data_type=Boolean  arith_type=Boolean  n_bits=1  bin_pt=0  float_type=Single  exp_bits=8  fraction_bits=24  quantization=Truncate  overflow=Wrap  en=off  latency=0  dbl_ovrd=off  pipeline=off  block_type=convert
  Register2 : xbsIndex_r4/Register  init=0  rst=on  en=on  dbl_ovrd=off  block_type=register
      settings: xl_area=[1 1 0 0 0 0 0]  block_version=VER_STRING_GOES_HERE
  Q : Outport
  wires:
  Convert2/1 -> Register2/3
  Convert1/1 -> Register2/2
  S/1 -> Convert2/1
  Register2/1 -> Q/1
  R/1 -> Convert1/1
  Constant1/1 -> Register2/1

== system wlan_phy_rx_pmd/Sync & Antenna Sel/LTS Correlation/S-R Latch1 ==
  S : Inport
  R : Inport  Port=2
  Constant1 : xbsIndex_r4/Constant  const=1  gui_display_data_type=Fixed-point  arith_type=Boolean  n_bits=1  bin_pt=0  preci_type=Single  exp_width=8  frac_width=24  explicit_period=off  period=1  equ=P=C  opselect=C  inp2=PCIN>>17  opr=+  inp1=P  carry=CIN  dbl_ovrd=off  block_type=constant
      settings: block_version=VER_STRING_GOES_HERE
  Convert1 : xbsIndex_r4/Convert  gui_display_data_type=Boolean  arith_type=Boolean  n_bits=1  bin_pt=0  float_type=Single  exp_bits=8  fraction_bits=24  quantization=Truncate  overflow=Wrap  en=off  latency=0  dbl_ovrd=off  pipeline=off  block_type=convert
  Convert2 : xbsIndex_r4/Convert  gui_display_data_type=Boolean  arith_type=Boolean  n_bits=1  bin_pt=0  float_type=Single  exp_bits=8  fraction_bits=24  quantization=Truncate  overflow=Wrap  en=off  latency=0  dbl_ovrd=off  pipeline=off  block_type=convert
  Register2 : xbsIndex_r4/Register  init=0  rst=on  en=on  dbl_ovrd=off  block_type=register
      settings: xl_area=[1 1 0 0 0 0 0]  block_version=VER_STRING_GOES_HERE
  Q : Outport
  wires:
  Constant1/1 -> Register2/1
  R/1 -> Convert1/1
  Register2/1 -> Q/1
  S/1 -> Convert2/1
  Convert1/1 -> Register2/2
  Convert2/1 -> Register2/3

== system wlan_phy_rx_pmd/Sync & Antenna Sel/LTS Correlation/Threshold Select ==
  Pkt Det : Inport
  RSSI : Inport  Port=2
  Mux : xbsIndex_r4/Mux  inputs=2  en=off  latency=0  precision=Full  arith_type=Unsigned  n_bits=16  bin_pt=14  quantization=Truncate  overflow=Wrap  dbl_ovrd=off  block_type=mux
  Register1 : xbsIndex_r4/Register  init=0  rst=off  en=on  dbl_ovrd=off  block_type=register
  Relational1 : xbsIndex_r4/Relational  mode=a>b  en=off  latency=0  dbl_ovrd=off  block_type=relational
      settings: xl_area=[10 0 0 19 0 0 0]  block_version=VER_STRING_GOES_HERE
  posedge : Subsystem
  Corr Thresh : Outport
  wires:
  Relational1/1 -> Mux/1
  RSSI/1 -> Register1/1
  posedge/1(Q) -> Register1/2
  Register1/1 -> Relational1/1
  [regRx_LongCorr_RSSI_Thresh] -> Relational1/2
  [regRx_LongCorr_Thresh_highSNR] -> Mux/3
  [regRx_LongCorr_Thresh_lowSNR] -> Mux/2
  Pkt Det/1 -> posedge/1(D)
  Mux/1 -> Corr Thresh/1

== system wlan_phy_rx_pmd/Sync & Antenna Sel/PHY CCA/PHY CCA Logic ==
  note: Two modes for physical carrier sensing:
      | Mode 0: Any enabled antenna can assert BUSY if its RSSI exceeds the threshold
      | Mode 1: BUSY only if *all* enabled antennas exceed the threshold
  note: This 4-in OR deasserts BUSY
      | if all antennas are disabled.
  Det A : Inport
  Det B : Inport  Port=2
  Det C : Inport  Port=3
  Det D : Inport  Port=4
  Inverter{,1..3} (4 alike) : xbsIndex_r4/Inverter  en=off  latency=0  dbl_ovrd=off  block_type=inv
  Logical{,2..4} (4 alike) : xbsIndex_r4/Logical  logical_function=AND  inputs=2  en=off  latency=0  precision=Full  arith_type=Unsigned  n_bits=16  bin_pt=0  align_bp=on  dbl_ovrd=off  block_type=logical
  Logical1 : xbsIndex_r4/Logical  logical_function=OR  inputs=4  en=off  latency=0  precision=Full  arith_type=Unsigned  n_bits=16  bin_pt=0  align_bp=on  dbl_ovrd=off  block_type=logical
  Logical10 : xbsIndex_r4/Logical  logical_function=OR  inputs=4  en=off  latency=0  precision=Full  arith_type=Unsigned  n_bits=16  bin_pt=0  align_bp=on  dbl_ovrd=off  block_type=logical
  Logical5 : xbsIndex_r4/Logical  logical_function=AND  inputs=5  en=off  latency=0  precision=Full  arith_type=Unsigned  n_bits=16  bin_pt=0  align_bp=on  dbl_ovrd=off  block_type=logical
  Logical{6..9} (4 alike) : xbsIndex_r4/Logical  logical_function=OR  inputs=2  en=off  latency=0  precision=Full  arith_type=Unsigned  n_bits=16  bin_pt=0  align_bp=on  dbl_ovrd=off  block_type=logical
  Mux : xbsIndex_r4/Mux  inputs=2  en=off  latency=0  precision=Full  arith_type=Unsigned  n_bits=16  bin_pt=14  quantization=Truncate  overflow=Wrap  dbl_ovrd=off  block_type=mux
  BUSY : Outport
  wires:
  [regRx_pktDetEn_RFA] -> Logical/1
  Logical/1 -> Logical1/1, Logical6/1
  Logical2/1 -> Logical1/2, Logical7/1
  Logical1/1 -> Mux/2
  Det A/1 -> Logical/2
  Det B/1 -> Logical2/2
  [regRx_pktDetEn_RFB] -> Logical2/1
  Det C/1 -> Logical3/2
  Det D/1 -> Logical4/2
  [regRx_pktDetEn_RFC] -> Logical3/1
  [regRx_pktDetEn_RFD] -> Logical4/1
  Logical3/1 -> Logical1/3, Logical8/1
  Logical4/1 -> Logical1/4, Logical9/1
  Inverter/1 -> Logical6/2
  [regRx_pktDetEn_RFA] -> Inverter/1, Logical10/1
  Logical6/1 -> Logical5/1
  Logical7/1 -> Logical5/2
  [regRx_pktDetEn_RFB] -> Inverter1/1, Logical10/2
  Inverter1/1 -> Logical7/2
  Logical8/1 -> Logical5/3
  [regRx_pktDetEn_RFC] -> Inverter2/1, Logical10/3
  Inverter2/1 -> Logical8/2
  [regRx_pktDetEn_RFD] -> Inverter3/1, Logical10/4
  Inverter3/1 -> Logical9/2
  Logical9/1 -> Logical5/4
  Logical10/1 -> Logical5/5
  Logical5/1 -> Mux/3
  Mux/1 -> BUSY/1
  [regRx_phyCCA_Mode_Sel] -> Mux/1

== system wlan_phy_rx_pmd/Sync & Antenna Sel/PHY CCA/Rate-Length BUSY ==
  note: CCA_BUSY for any Rx pkt with valid SIGNAL field
      | Rx Active drops after last data-bearing sample plus
      | programmable extension. This block doesn't care about
      | actual decoding latency or supported rates.
      |
      | This block starts counting before the SIGNAL field is
      | decoded. If the SIGNAL field is invalid, the default rate/length
      | (24 bits @ 6Mbps) will be used. This will de-assert busy after
      | the symbols containing the undecodable SIGNAL field.
  note: Force reset if SIGNAL or HT-SIG is invalid, indicating
      | PHY header could not be intepretted for waveform
      | duration.
      |
      | Any other Rx event will keep this block running for
      | the duration of the Rx waveform, indepedent of the
      | Rx PHY pipeline's attempt to decode the waveform.
      | This keeps the MAC in sync with the medium by
      | aligning RX_END to the end of the waveform, even
      | for waveforms recevied below the physical CS
      | threshold.
  IQ Valid : Inport
  Start : Inport  Port=2
  Bits : Subsystem
  Bus Creator : BusCreator  Inputs=2
  Delay : xbsIndex_r4/Delay  rst=off  infoeditControl=Selection of Reset will increase slice count due to use of real FFs and instead of SRLs  en=off  latency=1  dbl_ovrd=off  reg_retiming=off  block_type=delay
  Extension : Subsystem
  Gateway Out{1..6,8} (7 alike) : xbsIndex_r4/Gateway Out  inherit_from_input=off  hdl_port=off  timing_constraint=None  locs_specified=off  LOCs={}  UseAsDAC=off  DACChannel='1'  block_type=gatewayout
  Logical1 : xbsIndex_r4/Logical  logical_function=OR  inputs=2  en=off  latency=0  precision=Full  arith_type=Unsigned  n_bits=16  bin_pt=0  align_bp=on  dbl_ovrd=off  block_type=logical
  Logical2 : xbsIndex_r4/Logical  logical_function=OR  inputs=2  en=off  latency=0  precision=Full  arith_type=Unsigned  n_bits=16  bin_pt=0  align_bp=on  dbl_ovrd=off  block_type=logical
  Logical3 : xbsIndex_r4/Logical  logical_function=OR  inputs=4  en=off  latency=0  precision=Full  arith_type=Unsigned  n_bits=16  bin_pt=0  align_bp=on  dbl_ovrd=off  block_type=logical
  OFDM Syms : Subsystem
  PHY Rx Busy : Scope  NumInputPorts=7
      settings: TimeRange=11300  YMin=-0.2~-1~-1~-1~-1~-1~-1  YMax=0.25~1~1~1~1~1~1  SaveName=ScopeData30
  Pkt Done : Outport
  wires:
  Gateway Out4/1 -> PHY Rx Busy/2  "Start"
  Gateway Out1/1 -> PHY Rx Busy/3  "Sym Inc"
  Gateway Out2/1 -> PHY Rx Busy/4  "Bits Done"
  IQ Valid/1 -> OFDM Syms/2(IQ valid), Extension/2(IQ Valid)
  Gateway Out8/1 -> PHY Rx Busy/5, Bus Creator/2  "Pkt Done"
  Start/1 -> Gateway Out4/1, Delay/1, Logical3/1
  OFDM Syms/1(Sym) -> Bits/1(Sym Inc), Gateway Out1/1
  Extension/1(Pkt Done) -> Gateway Out8/1, Logical1/2, Logical2/1
  Bits/1(Done) -> Extension/1(Bits Done), Gateway Out2/1
  [CS_ADC_I] -> Gateway Out3/1
  Gateway Out3/1 -> Bus Creator/1  "ADC I"
  Logical3/1 -> Bits/2(Rst), Gateway Out5/1, Extension/3(Rst), Logical2/2, Logical1/1
  [OFDM_RX_SIGNAL_INVALID] -> Logical3/2
  Gateway Out5/1 -> PHY Rx Busy/6  "Reset"
  Logical1/1 -> OFDM Syms/3(Rst)
  [HT_SIG_ERROR] -> Logical3/3, Gateway Out6/1
  [RX_GLOBAL_RESET] -> Logical3/4
  Bus Creator/1 -> PHY Rx Busy/1
  Gateway Out6/1 -> PHY Rx Busy/7  "HTSIG Error"
  Delay/1 -> OFDM Syms/1(Start)
  Logical2/1 -> Pkt Done/1

== system wlan_phy_rx_pmd/Sync & Antenna Sel/PHY CCA/S-R Latch1 ==
  S : Inport
  R : Inport  Port=2
  Constant1 : xbsIndex_r4/Constant  const=1  gui_display_data_type=Fixed-point  arith_type=Boolean  n_bits=1  bin_pt=0  preci_type=Single  exp_width=8  frac_width=24  explicit_period=off  period=1  equ=P=C  opselect=C  inp2=PCIN>>17  opr=+  inp1=P  carry=CIN  dbl_ovrd=off  block_type=constant
      settings: block_version=VER_STRING_GOES_HERE
  Convert1 : xbsIndex_r4/Convert  gui_display_data_type=Boolean  arith_type=Boolean  n_bits=1  bin_pt=0  float_type=Single  exp_bits=8  fraction_bits=24  quantization=Truncate  overflow=Wrap  en=off  latency=0  dbl_ovrd=off  pipeline=off  block_type=convert
  Convert2 : xbsIndex_r4/Convert  gui_display_data_type=Boolean  arith_type=Boolean  n_bits=1  bin_pt=0  float_type=Single  exp_bits=8  fraction_bits=24  quantization=Truncate  overflow=Wrap  en=off  latency=0  dbl_ovrd=off  pipeline=off  block_type=convert
  Register2 : xbsIndex_r4/Register  init=0  rst=on  en=on  dbl_ovrd=off  block_type=register
      settings: xl_area=[1 1 0 0 0 0 0]  block_version=VER_STRING_GOES_HERE
  Q : Outport
  wires:
  Constant1/1 -> Register2/1
  R/1 -> Convert1/1
  Register2/1 -> Q/1
  S/1 -> Convert2/1
  Convert1/1 -> Register2/2
  Convert2/1 -> Register2/3
